simulink model "V2x_TC_TrafficLights_cs"  (Simulink 7.6)
settings: Solver=ode5  SolverMode=SingleTasking  StartTime=0.0  StopTime=38  FixedStep=1/100  RelTol=1e-3
PreLoadFcn:
  | check_NUTS_defined
  | 
  | assignin('base',['model_closed_' bdroot],false);addpath('../includes_V2x');
InitFcn:
  | PreScanExperimentInitFcn(bdroot)
StopFcn:
  | PreScanExperimentStopFcn(bdroot);
  | 
undefined symbols (not defined in the file or in its folder): acceleration, actorID, actorType, DiscIntNeverNeededParam, GPS_alt, GPS_lat, GPS_long, intmax, lightBarInUse, messageID, Newton, publicActorType, Raphson, rotZ, sigbuilder_block, sireneInUse, trafficLightPriority, turnAdvice, velocity, x_ini_VRML, y_ini_VRML, yawRate, z_ini_VRML
libraries: simulink x172, PreScanSimulinkLibrary x20, PreScanUsefulBlocks x11, simulink_extras x9, simulink_need_slupdate x1
(part 5 of 27)

== system V2x_TC_TrafficLights_cs/TrafficSignPoleNL_2 ==
  Antenna_Tx_9_BusAssignment : BusAssignment  Tag=BusAntennaT  AssignedSignals=warning,messageID,actorID,actorType,trafficLightPriority,GPS_lat,GPS_long,GPS_alt,rotZ
  Antenna_Tx_9_Mux : Subsystem  Tag=MuxAntennaT  mask(PreScan Mux Block:){Channel=1, TimeStep=1/100}
      mask prompts: TimeStep "Time Step [s]"
      settings: DeleteFcn=PreScanBlockDeleteFcn;
      mask Display:
      | fprintf('Channel: %d',Channel)
      | port_label('input', 1, 'Warning signal ID')
      | port_label('input', 2, 'Maneuvre ID')
      | port_label('input', 3, 'Vehicle Type')
      | port_label('input', 4, 'Braking Capacity')
      | port_label('input', 5, 'Vehicle Size')
      | port_label('input', 6, 'spare')
  CAM generation time step : Constant  Value=0.1
  CAM repetition time step : Constant  Value=0.05
  Cartesian to GPS : Subsystem
  Green light : Constant  Value=0
  Ground_{1..5} (5 alike) : Ground
  Infrastructure CAM generator : Subsystem
  Orange light : Constant  Value=0
  Red light : Constant  Value=0
  X : Constant  Value=113.310546875
  Y : Constant  Value=87.223381042480469
  Z : Constant  Value=0
  actorID : Constant  Value=7
  actorType : Constant  Value=14
  messageID : Constant  Value=902
  rotZ : Constant  Value=90
  warning : Constant  Value=0
  Antenna_Tx_9 : Outport  Tag=AntennaTOutport
  wires:
  CAM generation time step/1 -> Infrastructure CAM generator/1(CAM gen time step)
  CAM repetition time step/1 -> Infrastructure CAM generator/2(CAM rep time step)
  warning/1 -> Infrastructure CAM generator/3(warning)
  messageID/1 -> Infrastructure CAM generator/4(messageID)
  actorID/1 -> Infrastructure CAM generator/5(actorID)
  actorType/1 -> Infrastructure CAM generator/6(actorType)
  Red light/1 -> Infrastructure CAM generator/7(Red light)
  Orange light/1 -> Infrastructure CAM generator/8(Orange light)
  Green light/1 -> Infrastructure CAM generator/9(Green light)
  rotZ/1 -> Infrastructure CAM generator/13(rotZ)
  Cartesian to GPS/1(lat) -> Infrastructure CAM generator/10(GPS_lat)
  Cartesian to GPS/2(long) -> Infrastructure CAM generator/11(GPS_long)
  Cartesian to GPS/3(alt) -> Infrastructure CAM generator/12(GPS_alt)
  X/1 -> Cartesian to GPS/1(X)
  Y/1 -> Cartesian to GPS/2(Y)
  Z/1 -> Cartesian to GPS/3(Z)
  Antenna_Tx_9_Mux/2(Out2) -> [Antenna_Tx_9]
  [Antenna_Tx_9] -> Antenna_Tx_9_BusAssignment/1
  Antenna_Tx_9_BusAssignment/1 -> Antenna_Tx_9_Mux/6(spare)
  Antenna_Tx_9_Mux/1(Out1) -> Antenna_Tx_9/1
  Infrastructure CAM generator/9(CAM_rotZ) -> Antenna_Tx_9_BusAssignment/10
  Infrastructure CAM generator/8(CAM_GPSalt) -> Antenna_Tx_9_BusAssignment/9
  Infrastructure CAM generator/7(CAM_GPSlong) -> Antenna_Tx_9_BusAssignment/8
  Infrastructure CAM generator/6(CAM_GPSlat) -> Antenna_Tx_9_BusAssignment/7
  Infrastructure CAM generator/5(CAM_trafficLightPriority) -> Antenna_Tx_9_BusAssignment/6
  Infrastructure CAM generator/4(CAM_vehicleType) -> Antenna_Tx_9_BusAssignment/5
  Infrastructure CAM generator/3(CAM_vehicleID) -> Antenna_Tx_9_BusAssignment/4
  Infrastructure CAM generator/2(CAM_messageID) -> Antenna_Tx_9_BusAssignment/3
  Infrastructure CAM generator/1(CAM_warning) -> Antenna_Tx_9_BusAssignment/2
  Ground_1/1 -> Antenna_Tx_9_Mux/1(warning_signal_id)
  Ground_2/1 -> Antenna_Tx_9_Mux/2(maneuvre_id)
  Ground_3/1 -> Antenna_Tx_9_Mux/3(vehicle_type)
  Ground_4/1 -> Antenna_Tx_9_Mux/4(brakingcapacity)
  Ground_5/1 -> Antenna_Tx_9_Mux/5(vehicle_size)

== system V2x_TC_TrafficLights_cs/TrafficSignPoleNL_3 ==
  Antenna_Tx_10_BusAssignment : BusAssignment  Tag=BusAntennaT  AssignedSignals=warning,messageID,actorID,actorType,trafficLightPriority,GPS_lat,GPS_long,GPS_alt,rotZ
  Antenna_Tx_10_Mux : Subsystem  Tag=MuxAntennaT  mask(PreScan Mux Block:){Channel=1, TimeStep=1/100}
      mask prompts: TimeStep "Time Step [s]"
      settings: DeleteFcn=PreScanBlockDeleteFcn;
      mask Display:
      | fprintf('Channel: %d',Channel)
      | port_label('input', 1, 'Warning signal ID')
      | port_label('input', 2, 'Maneuvre ID')
      | port_label('input', 3, 'Vehicle Type')
      | port_label('input', 4, 'Braking Capacity')
      | port_label('input', 5, 'Vehicle Size')
      | port_label('input', 6, 'spare')
  CAM generation time step : Constant  Value=0.1
  CAM repetition time step : Constant  Value=0.05
  Cartesian to GPS : Subsystem
  Green light : Constant  Value=0
  Ground_{1..5} (5 alike) : Ground
  Infrastructure CAM generator : Subsystem
  Orange light : Constant  Value=0
  Red light : Constant  Value=0
  X : Constant  Value=121.9896240234375
  Y : Constant  Value=75.71380615234375
  Z : Constant  Value=0
  actorID : Constant  Value=7
  actorType : Constant  Value=14
  messageID : Constant  Value=902
  rotZ : Constant  Value=270
  warning : Constant  Value=0
  Antenna_Tx_10 : Outport  Tag=AntennaTOutport
  wires:
  CAM generation time step/1 -> Infrastructure CAM generator/1(CAM gen time step)
  CAM repetition time step/1 -> Infrastructure CAM generator/2(CAM rep time step)
  warning/1 -> Infrastructure CAM generator/3(warning)
  messageID/1 -> Infrastructure CAM generator/4(messageID)
  actorID/1 -> Infrastructure CAM generator/5(actorID)
  actorType/1 -> Infrastructure CAM generator/6(actorType)
  Red light/1 -> Infrastructure CAM generator/7(Red light)
  Orange light/1 -> Infrastructure CAM generator/8(Orange light)
  Green light/1 -> Infrastructure CAM generator/9(Green light)
  rotZ/1 -> Infrastructure CAM generator/13(rotZ)
  Cartesian to GPS/1(lat) -> Infrastructure CAM generator/10(GPS_lat)
  Cartesian to GPS/2(long) -> Infrastructure CAM generator/11(GPS_long)
  Cartesian to GPS/3(alt) -> Infrastructure CAM generator/12(GPS_alt)
  X/1 -> Cartesian to GPS/1(X)
  Y/1 -> Cartesian to GPS/2(Y)
  Z/1 -> Cartesian to GPS/3(Z)
  Antenna_Tx_10_Mux/2(Out2) -> [Antenna_Tx_10]
  [Antenna_Tx_10] -> Antenna_Tx_10_BusAssignment/1
  Antenna_Tx_10_BusAssignment/1 -> Antenna_Tx_10_Mux/6(spare)
  Antenna_Tx_10_Mux/1(Out1) -> Antenna_Tx_10/1
  Infrastructure CAM generator/9(CAM_rotZ) -> Antenna_Tx_10_BusAssignment/10
  Infrastructure CAM generator/8(CAM_GPSalt) -> Antenna_Tx_10_BusAssignment/9
  Infrastructure CAM generator/7(CAM_GPSlong) -> Antenna_Tx_10_BusAssignment/8
  Infrastructure CAM generator/6(CAM_GPSlat) -> Antenna_Tx_10_BusAssignment/7
  Infrastructure CAM generator/5(CAM_trafficLightPriority) -> Antenna_Tx_10_BusAssignment/6
  Infrastructure CAM generator/4(CAM_vehicleType) -> Antenna_Tx_10_BusAssignment/5
  Infrastructure CAM generator/3(CAM_vehicleID) -> Antenna_Tx_10_BusAssignment/4
  Infrastructure CAM generator/2(CAM_messageID) -> Antenna_Tx_10_BusAssignment/3
  Infrastructure CAM generator/1(CAM_warning) -> Antenna_Tx_10_BusAssignment/2
  Ground_1/1 -> Antenna_Tx_10_Mux/1(warning_signal_id)
  Ground_2/1 -> Antenna_Tx_10_Mux/2(maneuvre_id)
  Ground_3/1 -> Antenna_Tx_10_Mux/3(vehicle_type)
  Ground_4/1 -> Antenna_Tx_10_Mux/4(brakingcapacity)
  Ground_5/1 -> Antenna_Tx_10_Mux/5(vehicle_size)

== system V2x_TC_TrafficLights_cs/TrafficSignPoleNL_4 ==
  Antenna_Tx_7_BusAssignment : BusAssignment  Tag=BusAntennaT  AssignedSignals=warning,messageID,actorID,actorType,trafficLightPriority,GPS_lat,GPS_long,GPS_alt,rotZ
  Antenna_Tx_7_Mux : Subsystem  Tag=MuxAntennaT  mask(PreScan Mux Block:){Channel=1, TimeStep=1/100}
      mask prompts: TimeStep "Time Step [s]"
      settings: DeleteFcn=PreScanBlockDeleteFcn;
      mask Display:
      | fprintf('Channel: %d',Channel)
      | port_label('input', 1, 'Warning signal ID')
      | port_label('input', 2, 'Maneuvre ID')
      | port_label('input', 3, 'Vehicle Type')
      | port_label('input', 4, 'Braking Capacity')
      | port_label('input', 5, 'Vehicle Size')
      | port_label('input', 6, 'spare')
  CAM generation time step : Constant  Value=0.1
  CAM repetition time step : Constant  Value=0.05
  Cartesian to GPS : Subsystem
  Green light : Constant  Value=0
  Ground_{1..5} (5 alike) : Ground
  Infrastructure CAM generator : Subsystem
  Orange light : Constant  Value=0
  Red light : Constant  Value=0
  X : Constant  Value=135.64398193359375
  Y : Constant  Value=9.1701803207397461
  Z : Constant  Value=0
  actorID : Constant  Value=7
  actorType : Constant  Value=14
  messageID : Constant  Value=903
  rotZ : Constant  Value=180
  warning : Constant  Value=0
  Antenna_Tx_7 : Outport  Tag=AntennaTOutport
  wires:
  CAM generation time step/1 -> Infrastructure CAM generator/1(CAM gen time step)
  CAM repetition time step/1 -> Infrastructure CAM generator/2(CAM rep time step)
  warning/1 -> Infrastructure CAM generator/3(warning)
  messageID/1 -> Infrastructure CAM generator/4(messageID)
  actorID/1 -> Infrastructure CAM generator/5(actorID)
  actorType/1 -> Infrastructure CAM generator/6(actorType)
  Red light/1 -> Infrastructure CAM generator/7(Red light)
  Orange light/1 -> Infrastructure CAM generator/8(Orange light)
  Green light/1 -> Infrastructure CAM generator/9(Green light)
  rotZ/1 -> Infrastructure CAM generator/13(rotZ)
  Cartesian to GPS/1(lat) -> Infrastructure CAM generator/10(GPS_lat)
  Cartesian to GPS/2(long) -> Infrastructure CAM generator/11(GPS_long)
  Cartesian to GPS/3(alt) -> Infrastructure CAM generator/12(GPS_alt)
  X/1 -> Cartesian to GPS/1(X)
  Y/1 -> Cartesian to GPS/2(Y)
  Z/1 -> Cartesian to GPS/3(Z)
  Antenna_Tx_7_Mux/2(Out2) -> [Antenna_Tx_7]
  [Antenna_Tx_7] -> Antenna_Tx_7_BusAssignment/1
  Antenna_Tx_7_BusAssignment/1 -> Antenna_Tx_7_Mux/6(spare)
  Antenna_Tx_7_Mux/1(Out1) -> Antenna_Tx_7/1
  Infrastructure CAM generator/9(CAM_rotZ) -> Antenna_Tx_7_BusAssignment/10
  Infrastructure CAM generator/8(CAM_GPSalt) -> Antenna_Tx_7_BusAssignment/9
  Infrastructure CAM generator/7(CAM_GPSlong) -> Antenna_Tx_7_BusAssignment/8
  Infrastructure CAM generator/6(CAM_GPSlat) -> Antenna_Tx_7_BusAssignment/7
  Infrastructure CAM generator/5(CAM_trafficLightPriority) -> Antenna_Tx_7_BusAssignment/6
  Infrastructure CAM generator/4(CAM_vehicleType) -> Antenna_Tx_7_BusAssignment/5
  Infrastructure CAM generator/3(CAM_vehicleID) -> Antenna_Tx_7_BusAssignment/4
  Infrastructure CAM generator/2(CAM_messageID) -> Antenna_Tx_7_BusAssignment/3
  Infrastructure CAM generator/1(CAM_warning) -> Antenna_Tx_7_BusAssignment/2
  Ground_1/1 -> Antenna_Tx_7_Mux/1(warning_signal_id)
  Ground_2/1 -> Antenna_Tx_7_Mux/2(maneuvre_id)
  Ground_3/1 -> Antenna_Tx_7_Mux/3(vehicle_type)
  Ground_4/1 -> Antenna_Tx_7_Mux/4(brakingcapacity)
  Ground_5/1 -> Antenna_Tx_7_Mux/5(vehicle_size)

== system V2x_TC_TrafficLights_cs/TrafficSignPoleNL_5 ==
  Antenna_Tx_11_BusAssignment : BusAssignment  Tag=BusAntennaT  AssignedSignals=warning,messageID,actorID,actorType,trafficLightPriority,GPS_lat,GPS_long,GPS_alt,rotZ
  Antenna_Tx_11_Mux : Subsystem  Tag=MuxAntennaT  mask(PreScan Mux Block:){Channel=1, TimeStep=1/100}
      mask prompts: TimeStep "Time Step [s]"
      settings: DeleteFcn=PreScanBlockDeleteFcn;
      mask Display:
      | fprintf('Channel: %d',Channel)
      | port_label('input', 1, 'Warning signal ID')
      | port_label('input', 2, 'Maneuvre ID')
      | port_label('input', 3, 'Vehicle Type')
      | port_label('input', 4, 'Braking Capacity')
      | port_label('input', 5, 'Vehicle Size')
      | port_label('input', 6, 'spare')
  CAM generation time step : Constant  Value=0.1
  CAM repetition time step : Constant  Value=0.05
  Cartesian to GPS : Subsystem
  Green light : Constant  Value=0
  Ground_{1..5} (5 alike) : Ground
  Infrastructure CAM generator : Subsystem
  Orange light : Constant  Value=0
  Red light : Constant  Value=0
  X : Constant  Value=181.33143615722656
  Y : Constant  Value=63.34881591796875
  Z : Constant  Value=0
  actorID : Constant  Value=7
  actorType : Constant  Value=14
  messageID : Constant  Value=904
  rotZ : Constant  Value=90
  warning : Constant  Value=0
  Antenna_Tx_11 : Outport  Tag=AntennaTOutport
  wires:
  CAM generation time step/1 -> Infrastructure CAM generator/1(CAM gen time step)
  CAM repetition time step/1 -> Infrastructure CAM generator/2(CAM rep time step)
  warning/1 -> Infrastructure CAM generator/3(warning)
  messageID/1 -> Infrastructure CAM generator/4(messageID)
  actorID/1 -> Infrastructure CAM generator/5(actorID)
  actorType/1 -> Infrastructure CAM generator/6(actorType)
  Red light/1 -> Infrastructure CAM generator/7(Red light)
  Orange light/1 -> Infrastructure CAM generator/8(Orange light)
  Green light/1 -> Infrastructure CAM generator/9(Green light)
  rotZ/1 -> Infrastructure CAM generator/13(rotZ)
  Cartesian to GPS/1(lat) -> Infrastructure CAM generator/10(GPS_lat)
  Cartesian to GPS/2(long) -> Infrastructure CAM generator/11(GPS_long)
  Cartesian to GPS/3(alt) -> Infrastructure CAM generator/12(GPS_alt)
  X/1 -> Cartesian to GPS/1(X)
  Y/1 -> Cartesian to GPS/2(Y)
  Z/1 -> Cartesian to GPS/3(Z)
  Antenna_Tx_11_Mux/2(Out2) -> [Antenna_Tx_11]
  [Antenna_Tx_11] -> Antenna_Tx_11_BusAssignment/1
  Antenna_Tx_11_BusAssignment/1 -> Antenna_Tx_11_Mux/6(spare)
  Antenna_Tx_11_Mux/1(Out1) -> Antenna_Tx_11/1
  Infrastructure CAM generator/9(CAM_rotZ) -> Antenna_Tx_11_BusAssignment/10
  Infrastructure CAM generator/8(CAM_GPSalt) -> Antenna_Tx_11_BusAssignment/9
  Infrastructure CAM generator/7(CAM_GPSlong) -> Antenna_Tx_11_BusAssignment/8
  Infrastructure CAM generator/6(CAM_GPSlat) -> Antenna_Tx_11_BusAssignment/7
  Infrastructure CAM generator/5(CAM_trafficLightPriority) -> Antenna_Tx_11_BusAssignment/6
  Infrastructure CAM generator/4(CAM_vehicleType) -> Antenna_Tx_11_BusAssignment/5
  Infrastructure CAM generator/3(CAM_vehicleID) -> Antenna_Tx_11_BusAssignment/4
  Infrastructure CAM generator/2(CAM_messageID) -> Antenna_Tx_11_BusAssignment/3
  Infrastructure CAM generator/1(CAM_warning) -> Antenna_Tx_11_BusAssignment/2
  Ground_1/1 -> Antenna_Tx_11_Mux/1(warning_signal_id)
  Ground_2/1 -> Antenna_Tx_11_Mux/2(maneuvre_id)
  Ground_3/1 -> Antenna_Tx_11_Mux/3(vehicle_type)
  Ground_4/1 -> Antenna_Tx_11_Mux/4(brakingcapacity)
  Ground_5/1 -> Antenna_Tx_11_Mux/5(vehicle_size)

== system V2x_TC_TrafficLights_cs/TrafficSignPoleNL_6 ==
  Antenna_Tx_12_BusAssignment : BusAssignment  Tag=BusAntennaT  AssignedSignals=warning,messageID,actorID,actorType,trafficLightPriority,GPS_lat,GPS_long,GPS_alt,rotZ
  Antenna_Tx_12_Mux : Subsystem  Tag=MuxAntennaT  mask(PreScan Mux Block:){Channel=1, TimeStep=1/100}
      mask prompts: TimeStep "Time Step [s]"
      settings: DeleteFcn=PreScanBlockDeleteFcn;
      mask Display:
      | fprintf('Channel: %d',Channel)
      | port_label('input', 1, 'Warning signal ID')
      | port_label('input', 2, 'Maneuvre ID')
      | port_label('input', 3, 'Vehicle Type')
      | port_label('input', 4, 'Braking Capacity')
      | port_label('input', 5, 'Vehicle Size')
      | port_label('input', 6, 'spare')
  CAM generation time step : Constant  Value=0.1
  CAM repetition time step : Constant  Value=0.05
  Cartesian to GPS : Subsystem
  Green light : Constant  Value=0
  Ground_{1..5} (5 alike) : Ground
  Infrastructure CAM generator : Subsystem
  Orange light : Constant  Value=0
  Red light : Constant  Value=0
  X : Constant  Value=167.64527893066406
  Y : Constant  Value=85.7379379272461
  Z : Constant  Value=0
  actorID : Constant  Value=7
  actorType : Constant  Value=14
  messageID : Constant  Value=1030
  rotZ : Constant  Value=0
  warning : Constant  Value=0
  Antenna_Tx_12 : Outport  Tag=AntennaTOutport
  wires:
  CAM generation time step/1 -> Infrastructure CAM generator/1(CAM gen time step)
  CAM repetition time step/1 -> Infrastructure CAM generator/2(CAM rep time step)
  warning/1 -> Infrastructure CAM generator/3(warning)
  messageID/1 -> Infrastructure CAM generator/4(messageID)
  actorID/1 -> Infrastructure CAM generator/5(actorID)
  actorType/1 -> Infrastructure CAM generator/6(actorType)
  Red light/1 -> Infrastructure CAM generator/7(Red light)
  Orange light/1 -> Infrastructure CAM generator/8(Orange light)
  Green light/1 -> Infrastructure CAM generator/9(Green light)
  rotZ/1 -> Infrastructure CAM generator/13(rotZ)
  Cartesian to GPS/1(lat) -> Infrastructure CAM generator/10(GPS_lat)
  Cartesian to GPS/2(long) -> Infrastructure CAM generator/11(GPS_long)
  Cartesian to GPS/3(alt) -> Infrastructure CAM generator/12(GPS_alt)
  X/1 -> Cartesian to GPS/1(X)
  Y/1 -> Cartesian to GPS/2(Y)
  Z/1 -> Cartesian to GPS/3(Z)
  Antenna_Tx_12_Mux/2(Out2) -> [Antenna_Tx_12]
  [Antenna_Tx_12] -> Antenna_Tx_12_BusAssignment/1
  Antenna_Tx_12_BusAssignment/1 -> Antenna_Tx_12_Mux/6(spare)
  Antenna_Tx_12_Mux/1(Out1) -> Antenna_Tx_12/1
  Infrastructure CAM generator/9(CAM_rotZ) -> Antenna_Tx_12_BusAssignment/10
  Infrastructure CAM generator/8(CAM_GPSalt) -> Antenna_Tx_12_BusAssignment/9
  Infrastructure CAM generator/7(CAM_GPSlong) -> Antenna_Tx_12_BusAssignment/8
  Infrastructure CAM generator/6(CAM_GPSlat) -> Antenna_Tx_12_BusAssignment/7
  Infrastructure CAM generator/5(CAM_trafficLightPriority) -> Antenna_Tx_12_BusAssignment/6
  Infrastructure CAM generator/4(CAM_vehicleType) -> Antenna_Tx_12_BusAssignment/5
  Infrastructure CAM generator/3(CAM_vehicleID) -> Antenna_Tx_12_BusAssignment/4
  Infrastructure CAM generator/2(CAM_messageID) -> Antenna_Tx_12_BusAssignment/3
  Infrastructure CAM generator/1(CAM_warning) -> Antenna_Tx_12_BusAssignment/2
  Ground_1/1 -> Antenna_Tx_12_Mux/1(warning_signal_id)
  Ground_2/1 -> Antenna_Tx_12_Mux/2(maneuvre_id)
  Ground_3/1 -> Antenna_Tx_12_Mux/3(vehicle_type)
  Ground_4/1 -> Antenna_Tx_12_Mux/4(brakingcapacity)
  Ground_5/1 -> Antenna_Tx_12_Mux/5(vehicle_size)

== system V2x_TC_TrafficLights_cs/TrafficSignPoleNL_7 ==
  Antenna_Tx_13_BusAssignment : BusAssignment  Tag=BusAntennaT  AssignedSignals=warning,messageID,actorID,actorType,trafficLightPriority,GPS_lat,GPS_long,GPS_alt,rotZ
  Antenna_Tx_13_Mux : Subsystem  Tag=MuxAntennaT  mask(PreScan Mux Block:){Channel=1, TimeStep=1/100}
      mask prompts: TimeStep "Time Step [s]"
      settings: DeleteFcn=PreScanBlockDeleteFcn;
      mask Display:
      | fprintf('Channel: %d',Channel)
      | port_label('input', 1, 'Warning signal ID')
      | port_label('input', 2, 'Maneuvre ID')
      | port_label('input', 3, 'Vehicle Type')
      | port_label('input', 4, 'Braking Capacity')
      | port_label('input', 5, 'Vehicle Size')
      | port_label('input', 6, 'spare')
  CAM generation time step : Constant  Value=0.1
  CAM repetition time step : Constant  Value=0.05
  Cartesian to GPS : Subsystem
  Green light : Constant  Value=0
  Ground_{1..5} (5 alike) : Ground
  Infrastructure CAM generator : Subsystem
  Orange light : Constant  Value=0
  Red light : Constant  Value=0
  X : Constant  Value=135.72129821777344
  Y : Constant  Value=77.188400268554687
  Z : Constant  Value=0
  actorID : Constant  Value=7
  actorType : Constant  Value=14
  messageID : Constant  Value=1030
  rotZ : Constant  Value=180
  warning : Constant  Value=0
  Antenna_Tx_13 : Outport  Tag=AntennaTOutport
  wires:
  CAM generation time step/1 -> Infrastructure CAM generator/1(CAM gen time step)
  CAM repetition time step/1 -> Infrastructure CAM generator/2(CAM rep time step)
  warning/1 -> Infrastructure CAM generator/3(warning)
  messageID/1 -> Infrastructure CAM generator/4(messageID)
  actorID/1 -> Infrastructure CAM generator/5(actorID)
  actorType/1 -> Infrastructure CAM generator/6(actorType)
  Red light/1 -> Infrastructure CAM generator/7(Red light)
  Orange light/1 -> Infrastructure CAM generator/8(Orange light)
  Green light/1 -> Infrastructure CAM generator/9(Green light)
  rotZ/1 -> Infrastructure CAM generator/13(rotZ)
  Cartesian to GPS/1(lat) -> Infrastructure CAM generator/10(GPS_lat)
  Cartesian to GPS/2(long) -> Infrastructure CAM generator/11(GPS_long)
  Cartesian to GPS/3(alt) -> Infrastructure CAM generator/12(GPS_alt)
  X/1 -> Cartesian to GPS/1(X)
  Y/1 -> Cartesian to GPS/2(Y)
  Z/1 -> Cartesian to GPS/3(Z)
  Antenna_Tx_13_Mux/2(Out2) -> [Antenna_Tx_13]
  [Antenna_Tx_13] -> Antenna_Tx_13_BusAssignment/1
  Antenna_Tx_13_BusAssignment/1 -> Antenna_Tx_13_Mux/6(spare)
  Antenna_Tx_13_Mux/1(Out1) -> Antenna_Tx_13/1
  Infrastructure CAM generator/9(CAM_rotZ) -> Antenna_Tx_13_BusAssignment/10
  Infrastructure CAM generator/8(CAM_GPSalt) -> Antenna_Tx_13_BusAssignment/9
  Infrastructure CAM generator/7(CAM_GPSlong) -> Antenna_Tx_13_BusAssignment/8
  Infrastructure CAM generator/6(CAM_GPSlat) -> Antenna_Tx_13_BusAssignment/7
  Infrastructure CAM generator/5(CAM_trafficLightPriority) -> Antenna_Tx_13_BusAssignment/6
  Infrastructure CAM generator/4(CAM_vehicleType) -> Antenna_Tx_13_BusAssignment/5
  Infrastructure CAM generator/3(CAM_vehicleID) -> Antenna_Tx_13_BusAssignment/4
  Infrastructure CAM generator/2(CAM_messageID) -> Antenna_Tx_13_BusAssignment/3
  Infrastructure CAM generator/1(CAM_warning) -> Antenna_Tx_13_BusAssignment/2
  Ground_1/1 -> Antenna_Tx_13_Mux/1(warning_signal_id)
  Ground_2/1 -> Antenna_Tx_13_Mux/2(maneuvre_id)
  Ground_3/1 -> Antenna_Tx_13_Mux/3(vehicle_type)
  Ground_4/1 -> Antenna_Tx_13_Mux/4(brakingcapacity)
  Ground_5/1 -> Antenna_Tx_13_Mux/5(vehicle_size)

== system V2x_TC_TrafficLights_cs/TrafficSignPoleNL_8 ==
  Antenna_Tx_2_BusAssignment : BusAssignment  Tag=BusAntennaT  AssignedSignals=warning,messageID,actorID,actorType,trafficLightPriority,GPS_lat,GPS_long,GPS_alt,rotZ
  Antenna_Tx_2_Mux : Subsystem  Tag=MuxAntennaT  mask(PreScan Mux Block:){Channel=1, TimeStep=1/100}
      mask prompts: TimeStep "Time Step [s]"
      settings: DeleteFcn=PreScanBlockDeleteFcn;
      mask Display:
      | fprintf('Channel: %d',Channel)
      | port_label('input', 1, 'Warning signal ID')
      | port_label('input', 2, 'Maneuvre ID')
      | port_label('input', 3, 'Vehicle Type')
      | port_label('input', 4, 'Braking Capacity')
      | port_label('input', 5, 'Vehicle Size')
      | port_label('input', 6, 'spare')
  CAM generation time step : Constant  Value=0.1
  CAM repetition time step : Constant  Value=0.05
  Cartesian to GPS : Subsystem
  Green light : Constant  Value=0
  Ground_{1..5} (5 alike) : Ground
  Infrastructure CAM generator : Subsystem
  Orange light : Constant  Value=0
  Red light : Constant  Value=0
  X : Constant  Value=55
  Y : Constant  Value=9.24553394317627
  Z : Constant  Value=0
  actorID : Constant  Value=7
  actorType : Constant  Value=14
  messageID : Constant  Value=905
  rotZ : Constant  Value=180
  warning : Constant  Value=0
  Antenna_Tx_2 : Outport  Tag=AntennaTOutport
  wires:
  CAM generation time step/1 -> Infrastructure CAM generator/1(CAM gen time step)
  CAM repetition time step/1 -> Infrastructure CAM generator/2(CAM rep time step)
  warning/1 -> Infrastructure CAM generator/3(warning)
  messageID/1 -> Infrastructure CAM generator/4(messageID)
  actorID/1 -> Infrastructure CAM generator/5(actorID)
  actorType/1 -> Infrastructure CAM generator/6(actorType)
  Red light/1 -> Infrastructure CAM generator/7(Red light)
  Orange light/1 -> Infrastructure CAM generator/8(Orange light)
  Green light/1 -> Infrastructure CAM generator/9(Green light)
  rotZ/1 -> Infrastructure CAM generator/13(rotZ)
  Cartesian to GPS/1(lat) -> Infrastructure CAM generator/10(GPS_lat)
  Cartesian to GPS/2(long) -> Infrastructure CAM generator/11(GPS_long)
  Cartesian to GPS/3(alt) -> Infrastructure CAM generator/12(GPS_alt)
  X/1 -> Cartesian to GPS/1(X)
  Y/1 -> Cartesian to GPS/2(Y)
  Z/1 -> Cartesian to GPS/3(Z)
  Antenna_Tx_2_Mux/2(Out2) -> [Antenna_Tx_2]
  [Antenna_Tx_2] -> Antenna_Tx_2_BusAssignment/1
  Antenna_Tx_2_BusAssignment/1 -> Antenna_Tx_2_Mux/6(spare)
  Antenna_Tx_2_Mux/1(Out1) -> Antenna_Tx_2/1
  Infrastructure CAM generator/9(CAM_rotZ) -> Antenna_Tx_2_BusAssignment/10
  Infrastructure CAM generator/8(CAM_GPSalt) -> Antenna_Tx_2_BusAssignment/9
  Infrastructure CAM generator/7(CAM_GPSlong) -> Antenna_Tx_2_BusAssignment/8
  Infrastructure CAM generator/6(CAM_GPSlat) -> Antenna_Tx_2_BusAssignment/7
  Infrastructure CAM generator/5(CAM_trafficLightPriority) -> Antenna_Tx_2_BusAssignment/6
  Infrastructure CAM generator/4(CAM_vehicleType) -> Antenna_Tx_2_BusAssignment/5
  Infrastructure CAM generator/3(CAM_vehicleID) -> Antenna_Tx_2_BusAssignment/4
  Infrastructure CAM generator/2(CAM_messageID) -> Antenna_Tx_2_BusAssignment/3
  Infrastructure CAM generator/1(CAM_warning) -> Antenna_Tx_2_BusAssignment/2
  Ground_1/1 -> Antenna_Tx_2_Mux/1(warning_signal_id)
  Ground_2/1 -> Antenna_Tx_2_Mux/2(maneuvre_id)
  Ground_3/1 -> Antenna_Tx_2_Mux/3(vehicle_type)
  Ground_4/1 -> Antenna_Tx_2_Mux/4(brakingcapacity)
  Ground_5/1 -> Antenna_Tx_2_Mux/5(vehicle_size)

== system V2x_TC_TrafficLights_cs/TrafficSignPoleNL_9 ==
  Antenna_Tx_14_BusAssignment : BusAssignment  Tag=BusAntennaT  AssignedSignals=warning,messageID,actorID,actorType,trafficLightPriority,GPS_lat,GPS_long,GPS_alt,rotZ
  Antenna_Tx_14_Mux : Subsystem  Tag=MuxAntennaT  mask(PreScan Mux Block:){Channel=1, TimeStep=1/100}
      mask prompts: TimeStep "Time Step [s]"
      settings: DeleteFcn=PreScanBlockDeleteFcn;
      mask Display:
      | fprintf('Channel: %d',Channel)
      | port_label('input', 1, 'Warning signal ID')
      | port_label('input', 2, 'Maneuvre ID')
      | port_label('input', 3, 'Vehicle Type')
      | port_label('input', 4, 'Braking Capacity')
      | port_label('input', 5, 'Vehicle Size')
      | port_label('input', 6, 'spare')
  CAM generation time step : Constant  Value=0.1
  CAM repetition time step : Constant  Value=0.05
  Cartesian to GPS : Subsystem
  Green light : Constant  Value=0
  Ground_{1..5} (5 alike) : Ground
  Infrastructure CAM generator : Subsystem
  Orange light : Constant  Value=0
  Red light : Constant  Value=0
  X : Constant  Value=113.48127746582031
  Y : Constant  Value=60.186416625976562
  Z : Constant  Value=0
  actorID : Constant  Value=7
  actorType : Constant  Value=14
  messageID : Constant  Value=1040
  rotZ : Constant  Value=90
  warning : Constant  Value=0
  Antenna_Tx_14 : Outport  Tag=AntennaTOutport
  wires:
  CAM generation time step/1 -> Infrastructure CAM generator/1(CAM gen time step)
  CAM repetition time step/1 -> Infrastructure CAM generator/2(CAM rep time step)
  warning/1 -> Infrastructure CAM generator/3(warning)
  messageID/1 -> Infrastructure CAM generator/4(messageID)
  actorID/1 -> Infrastructure CAM generator/5(actorID)
  actorType/1 -> Infrastructure CAM generator/6(actorType)
  Red light/1 -> Infrastructure CAM generator/7(Red light)
  Orange light/1 -> Infrastructure CAM generator/8(Orange light)
  Green light/1 -> Infrastructure CAM generator/9(Green light)
  rotZ/1 -> Infrastructure CAM generator/13(rotZ)
  Cartesian to GPS/1(lat) -> Infrastructure CAM generator/10(GPS_lat)
  Cartesian to GPS/2(long) -> Infrastructure CAM generator/11(GPS_long)
  Cartesian to GPS/3(alt) -> Infrastructure CAM generator/12(GPS_alt)
  X/1 -> Cartesian to GPS/1(X)
  Y/1 -> Cartesian to GPS/2(Y)
  Z/1 -> Cartesian to GPS/3(Z)
  Antenna_Tx_14_Mux/2(Out2) -> [Antenna_Tx_14]
  [Antenna_Tx_14] -> Antenna_Tx_14_BusAssignment/1
  Antenna_Tx_14_BusAssignment/1 -> Antenna_Tx_14_Mux/6(spare)
  Antenna_Tx_14_Mux/1(Out1) -> Antenna_Tx_14/1
  Infrastructure CAM generator/9(CAM_rotZ) -> Antenna_Tx_14_BusAssignment/10
  Infrastructure CAM generator/8(CAM_GPSalt) -> Antenna_Tx_14_BusAssignment/9
  Infrastructure CAM generator/7(CAM_GPSlong) -> Antenna_Tx_14_BusAssignment/8
  Infrastructure CAM generator/6(CAM_GPSlat) -> Antenna_Tx_14_BusAssignment/7
  Infrastructure CAM generator/5(CAM_trafficLightPriority) -> Antenna_Tx_14_BusAssignment/6
  Infrastructure CAM generator/4(CAM_vehicleType) -> Antenna_Tx_14_BusAssignment/5
  Infrastructure CAM generator/3(CAM_vehicleID) -> Antenna_Tx_14_BusAssignment/4
  Infrastructure CAM generator/2(CAM_messageID) -> Antenna_Tx_14_BusAssignment/3
  Infrastructure CAM generator/1(CAM_warning) -> Antenna_Tx_14_BusAssignment/2
  Ground_1/1 -> Antenna_Tx_14_Mux/1(warning_signal_id)
  Ground_2/1 -> Antenna_Tx_14_Mux/2(maneuvre_id)
  Ground_3/1 -> Antenna_Tx_14_Mux/3(vehicle_type)
  Ground_4/1 -> Antenna_Tx_14_Mux/4(brakingcapacity)
  Ground_5/1 -> Antenna_Tx_14_Mux/5(vehicle_size)

== system V2x_TC_TrafficLights_cs/VISUCasingLights_TrafficLightRoadSideNL_1 ==
  CasingLights : Inport
  Demux4 : Demux  Outputs=3
  Saturation1 : Saturate  UpperLimit=1  LowerLimit=0
  Saturation2 : Saturate  UpperLimit=1  LowerLimit=0
  Saturation3 : Saturate  UpperLimit=1  LowerLimit=0
  conv_Green : Subsystem
  conv_Orange : Subsystem
  conv_Red : Subsystem
  visu_CasingGreen : Subsystem  mask(ToSensorWorld){PortName=visu_LightCasingGreen_0, PortIndex=3894, PortWidth=16, TimeStep=1/100}
      mask prompts: PortName "Port Name [-]"; PortIndex "Index [-]"; PortWidth "Port Dimensions [-]"; TimeStep "Upload Period [s]"
      mask help: Sends signal(s) from Simulink to the Sensor World
      mask Help:
      | Sends signal(s) from Simulink to the Sensor World.
      | 
      | - Port Name [-]: specifies the name of the output terminal of the Simulink block.
      | - Index [-]: specifies the start position in the output vector sent to the Sensor World
      | - Port dimensions [-]: specifies the width (number of scalars) of the output port
      | - Upload period [s]: specifies the upload period (in seconds) of the port.
      mask Display: port_label('input', 1, PortName)
  visu_CasingOrange : Subsystem  mask(ToSensorWorld){PortName=visu_LightCasingOrange_0, PortIndex=3910, PortWidth=16, TimeStep=1/100}
      mask prompts: PortName "Port Name [-]"; PortIndex "Index [-]"; PortWidth "Port Dimensions [-]"; TimeStep "Upload Period [s]"
      mask help: Sends signal(s) from Simulink to the Sensor World
      mask Help:
      | Sends signal(s) from Simulink to the Sensor World.
      | 
      | - Port Name [-]: specifies the name of the output terminal of the Simulink block.
      | - Index [-]: specifies the start position in the output vector sent to the Sensor World
      | - Port dimensions [-]: specifies the width (number of scalars) of the output port
      | - Upload period [s]: specifies the upload period (in seconds) of the port.
      mask Display: port_label('input', 1, PortName)
  visu_CasingRed : Subsystem  mask(ToSensorWorld){PortName=visu_LightCasingRed_0, PortIndex=3926, PortWidth=16, TimeStep=1/100}
      mask prompts: PortName "Port Name [-]"; PortIndex "Index [-]"; PortWidth "Port Dimensions [-]"; TimeStep "Upload Period [s]"
      mask help: Sends signal(s) from Simulink to the Sensor World
      mask Help:
      | Sends signal(s) from Simulink to the Sensor World.
      | 
      | - Port Name [-]: specifies the name of the output terminal of the Simulink block.
      | - Index [-]: specifies the start position in the output vector sent to the Sensor World
      | - Port dimensions [-]: specifies the width (number of scalars) of the output port
      | - Upload period [s]: specifies the upload period (in seconds) of the port.
      mask Display: port_label('input', 1, PortName)
  wires:
  CasingLights/1 -> Demux4/1
  Demux4/3 -> Saturation3/1
  Demux4/2 -> Saturation2/1
  Demux4/1 -> Saturation1/1
  Saturation1/1 -> conv_Red/1(on_off)
  Saturation2/1 -> conv_Orange/1(on_off)
  Saturation3/1 -> conv_Green/1(on_off)
  conv_Red/1(RGBcolor) -> visu_CasingRed/1(Y)
  conv_Orange/1(RGBcolor) -> visu_CasingOrange/1(Y)
  conv_Green/1(RGBcolor) -> visu_CasingGreen/1(Y)

== system V2x_TC_TrafficLights_cs/VISUCasingLights_TrafficLightRoadSideNL_2 ==
  CasingLights : Inport
  Demux4 : Demux  Outputs=3
  Saturation1 : Saturate  UpperLimit=1  LowerLimit=0
  Saturation2 : Saturate  UpperLimit=1  LowerLimit=0
  Saturation3 : Saturate  UpperLimit=1  LowerLimit=0
  conv_Green : Subsystem
  conv_Orange : Subsystem
  conv_Red : Subsystem
  visu_CasingGreen : Subsystem  mask(ToSensorWorld){PortName=visu_LightCasingGreen_0, PortIndex=4147, PortWidth=16, TimeStep=1/100}
      mask prompts: PortName "Port Name [-]"; PortIndex "Index [-]"; PortWidth "Port Dimensions [-]"; TimeStep "Upload Period [s]"
      mask help: Sends signal(s) from Simulink to the Sensor World
      mask Help:
      | Sends signal(s) from Simulink to the Sensor World.
      | 
      | - Port Name [-]: specifies the name of the output terminal of the Simulink block.
      | - Index [-]: specifies the start position in the output vector sent to the Sensor World
      | - Port dimensions [-]: specifies the width (number of scalars) of the output port
      | - Upload period [s]: specifies the upload period (in seconds) of the port.
      mask Display: port_label('input', 1, PortName)
  visu_CasingOrange : Subsystem  mask(ToSensorWorld){PortName=visu_LightCasingOrange_0, PortIndex=4163, PortWidth=16, TimeStep=1/100}
      mask prompts: PortName "Port Name [-]"; PortIndex "Index [-]"; PortWidth "Port Dimensions [-]"; TimeStep "Upload Period [s]"
      mask help: Sends signal(s) from Simulink to the Sensor World
      mask Help:
      | Sends signal(s) from Simulink to the Sensor World.
      | 
      | - Port Name [-]: specifies the name of the output terminal of the Simulink block.
      | - Index [-]: specifies the start position in the output vector sent to the Sensor World
      | - Port dimensions [-]: specifies the width (number of scalars) of the output port
      | - Upload period [s]: specifies the upload period (in seconds) of the port.
      mask Display: port_label('input', 1, PortName)
  visu_CasingRed : Subsystem  mask(ToSensorWorld){PortName=visu_LightCasingRed_0, PortIndex=4179, PortWidth=16, TimeStep=1/100}
      mask prompts: PortName "Port Name [-]"; PortIndex "Index [-]"; PortWidth "Port Dimensions [-]"; TimeStep "Upload Period [s]"
      mask help: Sends signal(s) from Simulink to the Sensor World
      mask Help:
      | Sends signal(s) from Simulink to the Sensor World.
      | 
      | - Port Name [-]: specifies the name of the output terminal of the Simulink block.
      | - Index [-]: specifies the start position in the output vector sent to the Sensor World
      | - Port dimensions [-]: specifies the width (number of scalars) of the output port
      | - Upload period [s]: specifies the upload period (in seconds) of the port.
      mask Display: port_label('input', 1, PortName)
  wires:
  CasingLights/1 -> Demux4/1
  Demux4/3 -> Saturation3/1
  Demux4/2 -> Saturation2/1
  Demux4/1 -> Saturation1/1
  Saturation1/1 -> conv_Red/1(on_off)
  Saturation2/1 -> conv_Orange/1(on_off)
  Saturation3/1 -> conv_Green/1(on_off)
  conv_Red/1(RGBcolor) -> visu_CasingRed/1(Y)
  conv_Orange/1(RGBcolor) -> visu_CasingOrange/1(Y)
  conv_Green/1(RGBcolor) -> visu_CasingGreen/1(Y)

== system V2x_TC_TrafficLights_cs/VISUCasingLights_TrafficLightRoadSideNL_3 ==
  CasingLights : Inport
  Demux4 : Demux  Outputs=3
  Saturation1 : Saturate  UpperLimit=1  LowerLimit=0
  Saturation2 : Saturate  UpperLimit=1  LowerLimit=0
  Saturation3 : Saturate  UpperLimit=1  LowerLimit=0
  conv_Green : Subsystem
  conv_Orange : Subsystem
  conv_Red : Subsystem
  visu_CasingGreen : Subsystem  mask(ToSensorWorld){PortName=visu_LightCasingGreen_0, PortIndex=4400, PortWidth=16, TimeStep=1/100}
      mask prompts: PortName "Port Name [-]"; PortIndex "Index [-]"; PortWidth "Port Dimensions [-]"; TimeStep "Upload Period [s]"
      mask help: Sends signal(s) from Simulink to the Sensor World
      mask Help:
      | Sends signal(s) from Simulink to the Sensor World.
      | 
      | - Port Name [-]: specifies the name of the output terminal of the Simulink block.
      | - Index [-]: specifies the start position in the output vector sent to the Sensor World
      | - Port dimensions [-]: specifies the width (number of scalars) of the output port
      | - Upload period [s]: specifies the upload period (in seconds) of the port.
      mask Display: port_label('input', 1, PortName)
  visu_CasingOrange : Subsystem  mask(ToSensorWorld){PortName=visu_LightCasingOrange_0, PortIndex=4416, PortWidth=16, TimeStep=1/100}
      mask prompts: PortName "Port Name [-]"; PortIndex "Index [-]"; PortWidth "Port Dimensions [-]"; TimeStep "Upload Period [s]"
      mask help: Sends signal(s) from Simulink to the Sensor World
      mask Help:
      | Sends signal(s) from Simulink to the Sensor World.
      | 
      | - Port Name [-]: specifies the name of the output terminal of the Simulink block.
      | - Index [-]: specifies the start position in the output vector sent to the Sensor World
      | - Port dimensions [-]: specifies the width (number of scalars) of the output port
      | - Upload period [s]: specifies the upload period (in seconds) of the port.
      mask Display: port_label('input', 1, PortName)
  visu_CasingRed : Subsystem  mask(ToSensorWorld){PortName=visu_LightCasingRed_0, PortIndex=4432, PortWidth=16, TimeStep=1/100}
      mask prompts: PortName "Port Name [-]"; PortIndex "Index [-]"; PortWidth "Port Dimensions [-]"; TimeStep "Upload Period [s]"
      mask help: Sends signal(s) from Simulink to the Sensor World
      mask Help:
      | Sends signal(s) from Simulink to the Sensor World.
      | 
      | - Port Name [-]: specifies the name of the output terminal of the Simulink block.
      | - Index [-]: specifies the start position in the output vector sent to the Sensor World
      | - Port dimensions [-]: specifies the width (number of scalars) of the output port
      | - Upload period [s]: specifies the upload period (in seconds) of the port.
      mask Display: port_label('input', 1, PortName)
  wires:
  CasingLights/1 -> Demux4/1
  Demux4/3 -> Saturation3/1
  Demux4/2 -> Saturation2/1
  Demux4/1 -> Saturation1/1
  Saturation1/1 -> conv_Red/1(on_off)
  Saturation2/1 -> conv_Orange/1(on_off)
  Saturation3/1 -> conv_Green/1(on_off)
  conv_Red/1(RGBcolor) -> visu_CasingRed/1(Y)
  conv_Orange/1(RGBcolor) -> visu_CasingOrange/1(Y)
  conv_Green/1(RGBcolor) -> visu_CasingGreen/1(Y)

== system V2x_TC_TrafficLights_cs/VISUCasingLights_TrafficLightRoadSideNL_4 ==
  CasingLights : Inport
  Demux4 : Demux  Outputs=3
  Saturation1 : Saturate  UpperLimit=1  LowerLimit=0
  Saturation2 : Saturate  UpperLimit=1  LowerLimit=0
  Saturation3 : Saturate  UpperLimit=1  LowerLimit=0
  conv_Green : Subsystem
  conv_Orange : Subsystem
  conv_Red : Subsystem
  visu_CasingGreen : Subsystem  mask(ToSensorWorld){PortName=visu_LightCasingGreen_0, PortIndex=4653, PortWidth=16, TimeStep=1/100}
      mask prompts: PortName "Port Name [-]"; PortIndex "Index [-]"; PortWidth "Port Dimensions [-]"; TimeStep "Upload Period [s]"
      mask help: Sends signal(s) from Simulink to the Sensor World
      mask Help:
      | Sends signal(s) from Simulink to the Sensor World.
      | 
      | - Port Name [-]: specifies the name of the output terminal of the Simulink block.
      | - Index [-]: specifies the start position in the output vector sent to the Sensor World
      | - Port dimensions [-]: specifies the width (number of scalars) of the output port
      | - Upload period [s]: specifies the upload period (in seconds) of the port.
      mask Display: port_label('input', 1, PortName)
  visu_CasingOrange : Subsystem  mask(ToSensorWorld){PortName=visu_LightCasingOrange_0, PortIndex=4669, PortWidth=16, TimeStep=1/100}
      mask prompts: PortName "Port Name [-]"; PortIndex "Index [-]"; PortWidth "Port Dimensions [-]"; TimeStep "Upload Period [s]"
      mask help: Sends signal(s) from Simulink to the Sensor World
      mask Help:
      | Sends signal(s) from Simulink to the Sensor World.
      | 
      | - Port Name [-]: specifies the name of the output terminal of the Simulink block.
      | - Index [-]: specifies the start position in the output vector sent to the Sensor World
      | - Port dimensions [-]: specifies the width (number of scalars) of the output port
      | - Upload period [s]: specifies the upload period (in seconds) of the port.
      mask Display: port_label('input', 1, PortName)
  visu_CasingRed : Subsystem  mask(ToSensorWorld){PortName=visu_LightCasingRed_0, PortIndex=4685, PortWidth=16, TimeStep=1/100}
      mask prompts: PortName "Port Name [-]"; PortIndex "Index [-]"; PortWidth "Port Dimensions [-]"; TimeStep "Upload Period [s]"
      mask help: Sends signal(s) from Simulink to the Sensor World
      mask Help:
      | Sends signal(s) from Simulink to the Sensor World.
      | 
      | - Port Name [-]: specifies the name of the output terminal of the Simulink block.
      | - Index [-]: specifies the start position in the output vector sent to the Sensor World
      | - Port dimensions [-]: specifies the width (number of scalars) of the output port
      | - Upload period [s]: specifies the upload period (in seconds) of the port.
      mask Display: port_label('input', 1, PortName)
  wires:
  CasingLights/1 -> Demux4/1
  Demux4/3 -> Saturation3/1
  Demux4/2 -> Saturation2/1
  Demux4/1 -> Saturation1/1
  Saturation1/1 -> conv_Red/1(on_off)
  Saturation2/1 -> conv_Orange/1(on_off)
  Saturation3/1 -> conv_Green/1(on_off)
  conv_Red/1(RGBcolor) -> visu_CasingRed/1(Y)
  conv_Orange/1(RGBcolor) -> visu_CasingOrange/1(Y)
  conv_Green/1(RGBcolor) -> visu_CasingGreen/1(Y)

== system V2x_TC_TrafficLights_cs/VISUCasingLights_TrafficLightRoadSideNL_5 ==
  CasingLights : Inport
  Demux4 : Demux  Outputs=3
  Saturation1 : Saturate  UpperLimit=1  LowerLimit=0
  Saturation2 : Saturate  UpperLimit=1  LowerLimit=0
  Saturation3 : Saturate  UpperLimit=1  LowerLimit=0
  conv_Green : Subsystem
  conv_Orange : Subsystem
  conv_Red : Subsystem
  visu_CasingGreen : Subsystem  mask(ToSensorWorld){PortName=visu_LightCasingGreen_0, PortIndex=4906, PortWidth=16, TimeStep=1/100}
      mask prompts: PortName "Port Name [-]"; PortIndex "Index [-]"; PortWidth "Port Dimensions [-]"; TimeStep "Upload Period [s]"
      mask help: Sends signal(s) from Simulink to the Sensor World
      mask Help:
      | Sends signal(s) from Simulink to the Sensor World.
      | 
      | - Port Name [-]: specifies the name of the output terminal of the Simulink block.
      | - Index [-]: specifies the start position in the output vector sent to the Sensor World
      | - Port dimensions [-]: specifies the width (number of scalars) of the output port
      | - Upload period [s]: specifies the upload period (in seconds) of the port.
      mask Display: port_label('input', 1, PortName)
  visu_CasingOrange : Subsystem  mask(ToSensorWorld){PortName=visu_LightCasingOrange_0, PortIndex=4922, PortWidth=16, TimeStep=1/100}
      mask prompts: PortName "Port Name [-]"; PortIndex "Index [-]"; PortWidth "Port Dimensions [-]"; TimeStep "Upload Period [s]"
      mask help: Sends signal(s) from Simulink to the Sensor World
      mask Help:
      | Sends signal(s) from Simulink to the Sensor World.
      | 
      | - Port Name [-]: specifies the name of the output terminal of the Simulink block.
      | - Index [-]: specifies the start position in the output vector sent to the Sensor World
      | - Port dimensions [-]: specifies the width (number of scalars) of the output port
      | - Upload period [s]: specifies the upload period (in seconds) of the port.
      mask Display: port_label('input', 1, PortName)
  visu_CasingRed : Subsystem  mask(ToSensorWorld){PortName=visu_LightCasingRed_0, PortIndex=4938, PortWidth=16, TimeStep=1/100}
      mask prompts: PortName "Port Name [-]"; PortIndex "Index [-]"; PortWidth "Port Dimensions [-]"; TimeStep "Upload Period [s]"
      mask help: Sends signal(s) from Simulink to the Sensor World
      mask Help:
      | Sends signal(s) from Simulink to the Sensor World.
      | 
      | - Port Name [-]: specifies the name of the output terminal of the Simulink block.
      | - Index [-]: specifies the start position in the output vector sent to the Sensor World
      | - Port dimensions [-]: specifies the width (number of scalars) of the output port
      | - Upload period [s]: specifies the upload period (in seconds) of the port.
      mask Display: port_label('input', 1, PortName)
  wires:
  CasingLights/1 -> Demux4/1
  Demux4/3 -> Saturation3/1
  Demux4/2 -> Saturation2/1
  Demux4/1 -> Saturation1/1
  Saturation1/1 -> conv_Red/1(on_off)
  Saturation2/1 -> conv_Orange/1(on_off)
  Saturation3/1 -> conv_Green/1(on_off)
  conv_Red/1(RGBcolor) -> visu_CasingRed/1(Y)
  conv_Orange/1(RGBcolor) -> visu_CasingOrange/1(Y)
  conv_Green/1(RGBcolor) -> visu_CasingGreen/1(Y)

== system V2x_TC_TrafficLights_cs/VISUCasingLights_TrafficLightRoadSideNL_6 ==
  CasingLights : Inport
  Demux4 : Demux  Outputs=3
  Saturation1 : Saturate  UpperLimit=1  LowerLimit=0
  Saturation2 : Saturate  UpperLimit=1  LowerLimit=0
  Saturation3 : Saturate  UpperLimit=1  LowerLimit=0
  conv_Green : Subsystem
  conv_Orange : Subsystem
  conv_Red : Subsystem
  visu_CasingGreen : Subsystem  mask(ToSensorWorld){PortName=visu_LightCasingGreen_0, PortIndex=5159, PortWidth=16, TimeStep=1/100}
      mask prompts: PortName "Port Name [-]"; PortIndex "Index [-]"; PortWidth "Port Dimensions [-]"; TimeStep "Upload Period [s]"
      mask help: Sends signal(s) from Simulink to the Sensor World
      mask Help:
      | Sends signal(s) from Simulink to the Sensor World.
      | 
      | - Port Name [-]: specifies the name of the output terminal of the Simulink block.
      | - Index [-]: specifies the start position in the output vector sent to the Sensor World
      | - Port dimensions [-]: specifies the width (number of scalars) of the output port
      | - Upload period [s]: specifies the upload period (in seconds) of the port.
      mask Display: port_label('input', 1, PortName)
  visu_CasingOrange : Subsystem  mask(ToSensorWorld){PortName=visu_LightCasingOrange_0, PortIndex=5175, PortWidth=16, TimeStep=1/100}
      mask prompts: PortName "Port Name [-]"; PortIndex "Index [-]"; PortWidth "Port Dimensions [-]"; TimeStep "Upload Period [s]"
      mask help: Sends signal(s) from Simulink to the Sensor World
      mask Help:
      | Sends signal(s) from Simulink to the Sensor World.
      | 
      | - Port Name [-]: specifies the name of the output terminal of the Simulink block.
      | - Index [-]: specifies the start position in the output vector sent to the Sensor World
      | - Port dimensions [-]: specifies the width (number of scalars) of the output port
      | - Upload period [s]: specifies the upload period (in seconds) of the port.
      mask Display: port_label('input', 1, PortName)
  visu_CasingRed : Subsystem  mask(ToSensorWorld){PortName=visu_LightCasingRed_0, PortIndex=5191, PortWidth=16, TimeStep=1/100}
      mask prompts: PortName "Port Name [-]"; PortIndex "Index [-]"; PortWidth "Port Dimensions [-]"; TimeStep "Upload Period [s]"
      mask help: Sends signal(s) from Simulink to the Sensor World
      mask Help:
      | Sends signal(s) from Simulink to the Sensor World.
      | 
      | - Port Name [-]: specifies the name of the output terminal of the Simulink block.
      | - Index [-]: specifies the start position in the output vector sent to the Sensor World
      | - Port dimensions [-]: specifies the width (number of scalars) of the output port
      | - Upload period [s]: specifies the upload period (in seconds) of the port.
      mask Display: port_label('input', 1, PortName)
  wires:
  CasingLights/1 -> Demux4/1
  Demux4/3 -> Saturation3/1
  Demux4/2 -> Saturation2/1
  Demux4/1 -> Saturation1/1
  Saturation1/1 -> conv_Red/1(on_off)
  Saturation2/1 -> conv_Orange/1(on_off)
  Saturation3/1 -> conv_Green/1(on_off)
  conv_Red/1(RGBcolor) -> visu_CasingRed/1(Y)
  conv_Orange/1(RGBcolor) -> visu_CasingOrange/1(Y)
  conv_Green/1(RGBcolor) -> visu_CasingGreen/1(Y)
